simulink model "TosAeb"  (R2021a)
settings: Solver=FixedStepDiscrete  StartTime=0.0  StopTime=inf  FixedStep=0.01  RelTol=1e-3
undefined symbols (not defined in the file or in its folder): ACCModSts, AccSts1, AEB_ObjectMovingOrientation, AEB_ObjectMovingState, CN_BTCActive, CN_CurrentGearR, CN_CurrentGearR_DHT, CN_PTCActive, CN_VDCActive, CN_VSC_EspOff, CN_VSC_FCW_AEB_Error, CN_VSC_FCW_AEB_NoDispay, CN_VSC_FCW_AEB_PerfmDgdtn, CN_VSC_FCW_AEB_TempUnAvlbl, CN_VSC_VehStandstill, CNaccelAvoidMax, CNaccelAvoidMin, CNbrakePedalPress, CNcomfortStrLeftAccelMax, CNcomfortStrLeftAccelMin, CNcomfortStrRightAccelMax, CNcomfortStrRightAccelMin, CNDrvDoorOpen, CNDrvModeEco, CNDrvModeNorm, CNDrvModeOther, CNDrvModeSport, CNDrvSBRreminder, CNNull, CNobjInvalidID, CNPassDoorOpen, CNPassSBRreminder, CNPEBSAccelNegInf, CNPEBSAccelNull, CNPEBSTimeInf, CNPEBSTimeNull, CNSTOPMAX, CNSTOPNULL, CNsubjWidth, CNSwtOFF, CNSwtON, CNTTCMAX, CNTTCNULL, CNVehConfigAEBCycNone, CNVehConfigAEBNone, CNVehConfigAEBPedNone, CNVehConfigFCWNone, CNVehConfigJANone, CollisionSeverity, FALSE, FCWSnvty, JACycObjCalcInfoDefaultParams, JAPedObjCalcInfoDefaultParams, JAVehObjCalcInfoDefaultParams, latCycObjCalcInfoDefaultParams, latPedObjCalcInfoDefaultParams, longObjCalcInfoDefaultParams, ModSts, movingStatus, ObjectType ... (+1112)
libraries: simulink x3031, AEBSFunctionLib x50, Ta_Library x33, AEBSStateMachineLib x23, Mylibrary x12, simulink_extras x7, ObjSelect_lib x3, dspstat3 x2
(part 14 of 102)

== system TosAeb/FcwAebJa_Step_sys/ALG_AEBSystem/ALG_AEBController/AEB_FuncCondCalcApp/LongFunctionCondCalc/vehAEBfullSuppAborCondCalc ==
  inputSignals : Inport
  " vehAEBfullSuppCondCalc" : Subsystem
  vehAEBfullAborCondCalc : Subsystem
  vehAEBfullSuppCond : Outport
  vehAEBfullAborCondNoStandstill : Outport  Port=2
  vehAEBfullAborCondStandstill : Outport  Port=3
  wires:
  vehAEBfullAborCondCalc/2(vehAEBfullAborCondStandstillFulfilled) -> vehAEBfullAborCondStandstill/1  "vehAEBfullAborCondStandstill"
  " vehAEBfullSuppCondCalc"/1(vehAEBfullSuppCondFulfilled) -> vehAEBfullSuppCond/1  "vehAEBfullSuppCond"
  vehAEBfullAborCondCalc/1(vehAEBfullAborCondNoStandstillFulfilled) -> vehAEBfullAborCondNoStandstill/1  "vehAEBfullAborCondNoStandstill"
  inputSignals/1 -> " vehAEBfullSuppCondCalc"/1(inputSignals), vehAEBfullAborCondCalc/1(inputSignals)

== system TosAeb/FcwAebJa_Step_sys/ALG_AEBSystem/ALG_AEBController/AEB_FuncCondCalcApp/LongFunctionCondCalc/vehAEBpartSuppAborCondCalc ==
  inputSignals : Inport
  vehAEBpartAborCondCalc : Subsystem
  vehAEBpartSuppCondCalc : Subsystem
  vehAEBpartSuppCond : Outport
  vehAEBpartAborCondNoStandstill : Outport  Port=2
  vehAEBpartAborCondStandstill : Outport  Port=3
  wires:
  vehAEBpartSuppCondCalc/1(vehAEBpartSuppCondFulfilled) -> vehAEBpartSuppCond/1  "vehAEBpartSuppCond"
  vehAEBpartAborCondCalc/2(vehAEBpartAborCondStandstillFulfilled) -> vehAEBpartAborCondStandstill/1  "vehAEBpartAborCondStandstill"
  vehAEBpartAborCondCalc/1(vehAEBpartAborCondNoStandstillFulfilled) -> vehAEBpartAborCondNoStandstill/1  "vehAEBpartAborCondNoStandstill"
  inputSignals/1 -> vehAEBpartSuppCondCalc/1(inputSignals), vehAEBpartAborCondCalc/1(inputSignals)

== system TosAeb/FcwAebJa_Step_sys/ALG_AEBSystem/ALG_AEBController/AEB_FuncCondCalcApp/LongFunctionCondCalc/vehAcuteWarningSuppAborCondFulfilled ==
  inputSignals : Inport
  vehAcuteWarningAborCondCalc : Subsystem
  vehAcuteWarningSuppCondCalc : Subsystem
  vehAcuteWarningSuppCond : Outport
  vehAcuteWarningAborCond : Outport  Port=2
  wires:
  vehAcuteWarningSuppCondCalc/1(vehAcuteWarningSuppCond) -> vehAcuteWarningSuppCond/1  "vehAcuteWarningSuppCond"
  vehAcuteWarningAborCondCalc/1(vehAcuteWarningAborCond) -> vehAcuteWarningAborCond/1  "vehAcuteWarningAborCond"
  inputSignals/1 -> vehAcuteWarningSuppCondCalc/1(inputSignals), vehAcuteWarningAborCondCalc/1(inputSignals)

== system TosAeb/FcwAebJa_Step_sys/ALG_AEBSystem/ALG_AEBController/AEB_FuncCondCalcApp/LongFunctionCondCalc/vehEBASuppAborCondCalc ==
  inputSignals : Inport
  vehEBAAborCondCalc : Subsystem
  vehEBASuppCondCalc : Subsystem
  vehEBASuppCond : Outport
  vehEBAAborCondNoStandstill : Outport  Port=2
  vehEBAAborCondStandstill : Outport  Port=3
  wires:
  vehEBASuppCondCalc/1(vehEBASuppCondFulfilled) -> vehEBASuppCond/1  "vehEBASuppCond"
  vehEBAAborCondCalc/1(vehEBAAborCondNoStandstillFulfilled) -> vehEBAAborCondNoStandstill/1  "vehEBAAborCondNoStandstill"
  vehEBAAborCondCalc/2(vehEBAAborCondStandstillFulfilled) -> vehEBAAborCondStandstill/1  "vehEBAAborCondStandstill"
  inputSignals/1 -> vehEBASuppCondCalc/1(inputSignals), vehEBAAborCondCalc/1(inputSignals)

== system TosAeb/FcwAebJa_Step_sys/ALG_AEBSystem/ALG_AEBController/AEB_FuncCondCalcApp/LongFunctionCondCalc/vehLatentWarningSuppAborCondFulfilled ==
  inputSignals : Inport
  vehLatentWarningAborCondCalc : Subsystem
  vehLatentWarningSuppCondCalc : Subsystem
  vehLatentWarningSuppCond : Outport
  vehLatentWarningAborCond : Outport  Port=2
  wires:
  vehLatentWarningAborCondCalc/1(vehLatentWarningAborCondFulfilled) -> vehLatentWarningAborCond/1  "vehLatentWarningAborCond"
  vehLatentWarningSuppCondCalc/1(vehLatentWarningSuppCondFulfilled) -> vehLatentWarningSuppCond/1  "vehLatentWarningSuppCond"
  inputSignals/1 -> vehLatentWarningAborCondCalc/1(inputSignals), vehLatentWarningSuppCondCalc/1(inputSignals)

== system TosAeb/FcwAebJa_Step_sys/ALG_AEBSystem/ALG_AEBController/AEB_FuncCondCalcApp/LongFunctionCondCalc/vehPreWarningSuppAborCondFulfilled ==
  inputSignals : Inport
  vehPreWarningAborCondCalc : Subsystem
  vehPreWarningSuppCondCalc : Subsystem
  vehPreWarningSuppCond : Outport
  vehPreWarningAborCond : Outport  Port=2
  wires:
  inputSignals/1 -> vehPreWarningSuppCondCalc/1(inputSignals), vehPreWarningAborCondCalc/1(inputSignals)
  vehPreWarningSuppCondCalc/1(vehPreWarningSuppCond) -> vehPreWarningSuppCond/1  "vehPreWarningSuppCond"
  vehPreWarningAborCondCalc/1(vehPreWarningAborCond) -> vehPreWarningAborCond/1  "vehPreWarningAborCond"

== system TosAeb/FcwAebJa_Step_sys/ALG_AEBSystem/ALG_AEBController/AEB_FuncCondCalcApp/LongFunctionCondCalc/vehPrefillSuppAborCondCalc ==
  inputSignals : Inport
  vehPrefillAborCondCalc : Subsystem
  vehPrefillSuppCondCalc : Subsystem
  vehPrefillSuppCond : Outport
  vehPrefillAborCond : Outport  Port=2
  wires:
  vehPrefillAborCondCalc/1(vehPrefillAborCondFulfilled) -> vehPrefillAborCond/1  "vehPrefillAborCond"
  vehPrefillSuppCondCalc/1(vehPrefillSuppCondFulfilled) -> vehPrefillSuppCond/1  "vehPrefillSuppCond"
  inputSignals/1 -> vehPrefillSuppCondCalc/1(inputSignals), vehPrefillAborCondCalc/1(inputSignals)

== system TosAeb/FcwAebJa_Step_sys/ALG_AEBSystem/ALG_AEBController/AEB_FuncCondCalcApp/PedFunctionCondCalc/pedABASuppAborCond ==
  inputSignals : Inport
  pedABAAborCondCalc : Subsystem
  pedABASuppCondCalc : Subsystem
  pedABASuppCond : Outport
  pedABAAborCond : Outport  Port=2
  wires:
  inputSignals/1 -> pedABASuppCondCalc/1(inputSignals), pedABAAborCondCalc/1(inputSignals)
  pedABASuppCondCalc/1(pedABASuppCondFulfilled) -> pedABASuppCond/1  "pedPreWarningSuppCond"
  pedABAAborCondCalc/1(pedABAAborCondFulfilled) -> pedABAAborCond/1  "pedPreWarningAborCond"

== system TosAeb/FcwAebJa_Step_sys/ALG_AEBSystem/ALG_AEBController/AEB_FuncCondCalcApp/PedFunctionCondCalc/pedAEBfullSuppAborCond ==
  inputSignals : Inport
  pedAEBfullAborCondNoStandstillCalc : Subsystem
  pedAEBfullAborCondStandstillCalc : Subsystem
  pedAEBfullSuppCondCalc : Subsystem
  pedAEBfullSuppCond : Outport
  pedAEBfullAborCondNoStandstill : Outport  Port=2
  pedAEBfullAborCondStandstill : Outport  Port=3
  wires:
  pedAEBfullSuppCondCalc/1(pedAEBfullSuppCondFulfilled) -> pedAEBfullSuppCond/1  "pedAEBfullSuppCond"
  pedAEBfullAborCondNoStandstillCalc/1(pedAEBfullAborCondNoStandstillFulfilled) -> pedAEBfullAborCondNoStandstill/1  "pedAEBfullAborCondNoStandstill"
  pedAEBfullAborCondStandstillCalc/1(pedAEBfullAborCondStandstillFulfilled) -> pedAEBfullAborCondStandstill/1  "pedAEBfullAborCondStandstill"
  inputSignals/1 -> pedAEBfullAborCondStandstillCalc/1(inputSignals), pedAEBfullAborCondNoStandstillCalc/1(inputSignals), pedAEBfullSuppCondCalc/1(inputSignals)

== system TosAeb/FcwAebJa_Step_sys/ALG_AEBSystem/ALG_AEBController/AEB_FuncCondCalcApp/PedFunctionCondCalc/pedPreWarningSuppAborCond ==
  inputSignals : Inport
  pedPreWarningAborCondCalc : Subsystem
  pedPreWarningSuppCondCalc : Subsystem
  pedPreWarningSuppCond : Outport
  pedPreWarningAborCond : Outport  Port=2
  wires:
  pedPreWarningAborCondCalc/1(pedPreWarningAborCondFulfilled) -> pedPreWarningAborCond/1  "pedPreWarningAborCond"
  pedPreWarningSuppCondCalc/1(pedPreWarningSuppCondFulfilled) -> pedPreWarningSuppCond/1  "pedPreWarningSuppCond"
  inputSignals/1 -> pedPreWarningAborCondCalc/1(inputSignals), pedPreWarningSuppCondCalc/1(inputSignals)

== system TosAeb/FcwAebJa_Step_sys/ALG_AEBSystem/ALG_AEBController/AEB_FuncCondCalcApp/PedFunctionCondCalc/pedPrefillSuppAborCond ==
  inputSignals : Inport
  pedPrefillAborCondCalc : Subsystem
  pedPrefillSuppCondCalc : Subsystem
  pedPrefillSuppCond : Outport
  pedPrefillAborCond : Outport  Port=2
  wires:
  inputSignals/1 -> pedPrefillSuppCondCalc/1(inputSignals), pedPrefillAborCondCalc/1(inputSignals)
  pedPrefillAborCondCalc/1(pedPrefillAborCondFulfilled) -> pedPrefillAborCond/1  "pedPrefillAborCond"
  pedPrefillSuppCondCalc/1(pedPrefillSuppCondFulfilled) -> pedPrefillSuppCond/1  "pedPrefillSuppCond"

== system TosAeb/FcwAebJa_Step_sys/ALG_AEBSystem/ALG_AEBController/AEB_FuncCondCalcInfo_FUS/CycFunctionCondCalc/cycAEBfullSuppAborCond ==
  inputSignals : Inport
  Bus Selector : BusSelector  OutputSignals=FuncCondCalcInfo.CycFuncSuppAborCond.cycAEBfullSuppCond
  Bus Selector1 : BusSelector  OutputSignals=FuncCondCalcInfo.CycFuncSuppAborCond.cycAEBfullAborCondStandstill
  Bus Selector2 : BusSelector  OutputSignals=FuncCondCalcInfo.CycFuncSuppAborCond.cycAEBfullAborCondNoStandstill
  Constant : Constant
  Constant1 : Constant
  Constant2 : Constant
  Multiport Switch : MultiPortSwitch
  Multiport Switch1 : MultiPortSwitch
  Multiport Switch2 : MultiPortSwitch
  cycAEBfullSuppCond : Outport
  cycAEBfullAborCondNoStandstill : Outport  Port=2
  cycAEBfullAborCondStandstill : Outport  Port=3
  wires:
  Bus Selector1/1 -> Multiport Switch1/4, Multiport Switch1/3, Multiport Switch1/2  "<cycAEBfullAborCondStandstill>"
  Constant1/1 -> Multiport Switch1/1
  Constant/1 -> Multiport Switch/1
  Bus Selector2/1 -> Multiport Switch2/4, Multiport Switch2/3, Multiport Switch2/2  "<cycAEBfullAborCondNoStandstill>"
  Constant2/1 -> Multiport Switch2/1
  Bus Selector/1 -> Multiport Switch/4, Multiport Switch/3, Multiport Switch/2  "<cycAEBfullSuppCond>"
  inputSignals/1 -> Bus Selector/1, Bus Selector1/1, Bus Selector2/1
  Multiport Switch1/1 -> cycAEBfullAborCondStandstill/1  "cycAEBfullAborCondStandstill"
  Multiport Switch2/1 -> cycAEBfullAborCondNoStandstill/1  "cycAEBfullAborCondNoStandstill"
  Multiport Switch/1 -> cycAEBfullSuppCond/1  "cycAEBfullSuppCond"

== system TosAeb/FcwAebJa_Step_sys/ALG_AEBSystem/ALG_AEBController/AEB_FuncCondCalcInfo_FUS/CycFunctionCondCalc/cycAEBpartSuppAborCond ==
  inputSignals : Inport
  Bus Selector : BusSelector  OutputSignals=FuncCondCalcInfo.CycFuncSuppAborCond.cycAEBpartSuppCond
  Bus Selector1 : BusSelector  OutputSignals=FuncCondCalcInfo.CycFuncSuppAborCond.cycAEBpartAborCondStandstill
  Bus Selector2 : BusSelector  OutputSignals=FuncCondCalcInfo.CycFuncSuppAborCond.cycAEBpartAborCondNoStandstill
  Constant : Constant
  Constant1 : Constant
  Constant2 : Constant
  Multiport Switch : MultiPortSwitch
  Multiport Switch1 : MultiPortSwitch
  Multiport Switch2 : MultiPortSwitch
  cycAEBpartSuppCond : Outport
  cycAEBpartAborCondNoStandstill : Outport  Port=2
  cycAEBpartAborCondStandstill : Outport  Port=3
  wires:
  Bus Selector1/1 -> Multiport Switch1/4, Multiport Switch1/3, Multiport Switch1/2  "<cycAEBpartAborCondStandstill>"
  Constant1/1 -> Multiport Switch1/1
  Constant/1 -> Multiport Switch/1
  Bus Selector2/1 -> Multiport Switch2/4, Multiport Switch2/3, Multiport Switch2/2  "<cycAEBpartAborCondNoStandstill>"
  Constant2/1 -> Multiport Switch2/1
  Bus Selector/1 -> Multiport Switch/4, Multiport Switch/3, Multiport Switch/2  "<cycAEBpartSuppCond>"
  Multiport Switch2/1 -> cycAEBpartAborCondNoStandstill/1  "cycAEBpartAborCondNoStandstill"
  Multiport Switch1/1 -> cycAEBpartAborCondStandstill/1  "cycAEBpartAborCondStandstill"
  inputSignals/1 -> Bus Selector1/1, Bus Selector2/1, Bus Selector/1
  Multiport Switch/1 -> cycAEBpartSuppCond/1  "cycAEBpartSuppCond"

== system TosAeb/FcwAebJa_Step_sys/ALG_AEBSystem/ALG_AEBController/AEB_FuncCondCalcInfo_FUS/CycFunctionCondCalc/cycPrefillSuppAborCond ==
  inputSignals : Inport
  Bus Selector : BusSelector  OutputSignals=FuncCondCalcInfo.CycFuncSuppAborCond.cycPrefillSuppCond
  Bus Selector1 : BusSelector  OutputSignals=FuncCondCalcInfo.CycFuncSuppAborCond.cycPrefillAborCond
  Constant : Constant
  Constant2 : Constant
  Multiport Switch : MultiPortSwitch
  Multiport Switch1 : MultiPortSwitch
  cycPrefillSuppCond : Outport
  cycPrefillAborCond : Outport  Port=2
  wires:
  inputSignals/1 -> Bus Selector1/1, Bus Selector/1
  Bus Selector/1 -> Multiport Switch/4, Multiport Switch/3, Multiport Switch/2  "<cycPrefillSuppCond>"
  Constant/1 -> Multiport Switch/1
  Constant2/1 -> Multiport Switch1/1
  Bus Selector1/1 -> Multiport Switch1/4, Multiport Switch1/3, Multiport Switch1/2  "<cycPrefillAborCond>"
  Multiport Switch/1 -> cycPrefillSuppCond/1  "cycPrefillSuppCond"
  Multiport Switch1/1 -> cycPrefillAborCond/1  "cycPrefillAborCond"

== system TosAeb/FcwAebJa_Step_sys/ALG_AEBSystem/ALG_AEBController/AEB_FuncCondCalcInfo_FUS/JaCycFunctionCondCalc/JaCycAEBfullSuppAborCond ==
  inputSignals : Inport
  Bus Selector : BusSelector  OutputSignals=FuncCondCalcInfo.JACycFuncSuppAborCond.JACycAEBfullSuppCond
  Bus Selector1 : BusSelector  OutputSignals=FuncCondCalcInfo.JACycFuncSuppAborCond.JACycAEBfullAborCondStandstill
  Bus Selector2 : BusSelector  OutputSignals=FuncCondCalcInfo.JACycFuncSuppAborCond.JACycAEBfullAborCondNoStandstill
  Constant : Constant
  Constant1 : Constant
  Constant2 : Constant
  Multiport Switch : MultiPortSwitch
  Multiport Switch1 : MultiPortSwitch
  Multiport Switch2 : MultiPortSwitch
  JaCycAEBfullSuppCond : Outport
  JaCycAEBfullAborCondNoStandstill : Outport  Port=2
  JaCycAEBfullAborCondStandstill : Outport  Port=3
  wires:
  Multiport Switch1/1 -> JaCycAEBfullAborCondStandstill/1  "AEBJAPedAEBfullAborCondStandstill"
  inputSignals/1 -> Bus Selector1/1, Bus Selector2/1, Bus Selector/1
  Multiport Switch2/1 -> JaCycAEBfullAborCondNoStandstill/1  "AEBJAPedAEBfullAborCondNoStandstill"
  Multiport Switch/1 -> JaCycAEBfullSuppCond/1  "AEBJAPedAEBfullSuppCond"
  Bus Selector/1 -> Multiport Switch/2, Multiport Switch/4, Multiport Switch/3  "<JACycAEBfullSuppCond>"
  Constant2/1 -> Multiport Switch2/1
  Bus Selector2/1 -> Multiport Switch2/4, Multiport Switch2/3, Multiport Switch2/2  "<JACycAEBfullAborCondNoStandstill>"
  Constant/1 -> Multiport Switch/1
  Constant1/1 -> Multiport Switch1/1
  Bus Selector1/1 -> Multiport Switch1/4, Multiport Switch1/3, Multiport Switch1/2  "<JACycAEBfullAborCondStandstill>"

== system TosAeb/FcwAebJa_Step_sys/ALG_AEBSystem/ALG_AEBController/AEB_FuncCondCalcInfo_FUS/JaCycFunctionCondCalc/JaCycPrefillSuppAborCond ==
  inputSignals : Inport
  Bus Selector : BusSelector  OutputSignals=FuncCondCalcInfo.JACycFuncSuppAborCond.JACycPrefillSuppCond
  Bus Selector1 : BusSelector  OutputSignals=FuncCondCalcInfo.JACycFuncSuppAborCond.JACycPrefillAborCond
  Constant : Constant
  Constant1 : Constant
  Multiport Switch : MultiPortSwitch
  Multiport Switch1 : MultiPortSwitch
  JaCycPrefillSuppCond : Outport
  JaCycPrefillAborCond : Outport  Port=2
  wires:
  Multiport Switch/1 -> JaCycPrefillSuppCond/1
  Multiport Switch1/1 -> JaCycPrefillAborCond/1
  Bus Selector1/1 -> Multiport Switch1/4, Multiport Switch1/3, Multiport Switch1/2  "<JACycPrefillAborCond>"
  Bus Selector/1 -> Multiport Switch/4, Multiport Switch/3, Multiport Switch/2  "<JACycPrefillSuppCond>"
  Constant1/1 -> Multiport Switch1/1
  Constant/1 -> Multiport Switch/1
  inputSignals/1 -> Bus Selector1/1, Bus Selector/1

== system TosAeb/FcwAebJa_Step_sys/ALG_AEBSystem/ALG_AEBController/AEB_FuncCondCalcInfo_FUS/JaPedFunctionCondCalc/AEBJAPedAEBfullSuppAborCond ==
  inputSignals : Inport
  Bus Selector : BusSelector  OutputSignals=FuncCondCalcInfo.JAPedFuncSuppAborCond.JAPedAEBfullSuppCond
  Bus Selector1 : BusSelector  OutputSignals=FuncCondCalcInfo.JAPedFuncSuppAborCond.JAPedAEBfullAborCondStandstill
  Bus Selector2 : BusSelector  OutputSignals=FuncCondCalcInfo.JAPedFuncSuppAborCond.JAPedAEBfullAborCondNoStandstill
  Constant : Constant
  Constant1 : Constant
  Constant2 : Constant
  Multiport Switch : MultiPortSwitch
  Multiport Switch1 : MultiPortSwitch
  Multiport Switch2 : MultiPortSwitch
  AEBJAPedAEBfullSuppCond : Outport
  AEBJAPedAEBfullAborCondNoStandstill : Outport  Port=2
  AEBJAPedAEBfullAborCondStandstill : Outport  Port=3
  wires:
  Bus Selector1/1 -> Multiport Switch1/2, Multiport Switch1/4, Multiport Switch1/3  "<JAPedAEBfullAborCondStandstill>"
  Constant1/1 -> Multiport Switch1/1
  Constant/1 -> Multiport Switch/1
  Bus Selector2/1 -> Multiport Switch2/2, Multiport Switch2/4, Multiport Switch2/3  "<JAPedAEBfullAborCondNoStandstill>"
  Constant2/1 -> Multiport Switch2/1
  Bus Selector/1 -> Multiport Switch/4, Multiport Switch/3, Multiport Switch/2  "<JAPedAEBfullSuppCond>"
  Multiport Switch/1 -> AEBJAPedAEBfullSuppCond/1  "AEBJAPedAEBfullSuppCond"
  Multiport Switch2/1 -> AEBJAPedAEBfullAborCondNoStandstill/1  "AEBJAPedAEBfullAborCondNoStandstill"
  inputSignals/1 -> Bus Selector1/1, Bus Selector2/1, Bus Selector/1
  Multiport Switch1/1 -> AEBJAPedAEBfullAborCondStandstill/1  "AEBJAPedAEBfullAborCondStandstill"

== system TosAeb/FcwAebJa_Step_sys/ALG_AEBSystem/ALG_AEBController/AEB_FuncCondCalcInfo_FUS/JaPedFunctionCondCalc/AEBJAPedPrefillSuppAborCond ==
  inputSignals : Inport
  Bus Selector : BusSelector  OutputSignals=FuncCondCalcInfo.JAPedFuncSuppAborCond.JAPedPrefillSuppCond
  Bus Selector1 : BusSelector  OutputSignals=FuncCondCalcInfo.JAPedFuncSuppAborCond.JAPedPrefillAborCond
  Constant : Constant
  Constant1 : Constant
  Multiport Switch : MultiPortSwitch
  Multiport Switch1 : MultiPortSwitch
  JaPedPrefillSuppCond : Outport
  JaPedPrefillAborCond : Outport  Port=2
  wires:
  inputSignals/1 -> Bus Selector1/1, Bus Selector/1
  Constant/1 -> Multiport Switch/1
  Constant1/1 -> Multiport Switch1/1
  Bus Selector/1 -> Multiport Switch/4, Multiport Switch/3, Multiport Switch/2  "<JAPedPrefillSuppCond>"
  Bus Selector1/1 -> Multiport Switch1/4, Multiport Switch1/3, Multiport Switch1/2  "<JAPedPrefillAborCond>"
  Multiport Switch1/1 -> JaPedPrefillAborCond/1  "AEBJAPedPrefillAborCond"
  Multiport Switch/1 -> JaPedPrefillSuppCond/1  "AEBJAPedPrefillSuppCond"

== system TosAeb/FcwAebJa_Step_sys/ALG_AEBSystem/ALG_AEBController/AEB_FuncCondCalcInfo_FUS/JaVehFunctionCondCalc/JaVehAEBfullSuppAborCond ==
  inputSignals : Inport
  Bus Selector : BusSelector  OutputSignals=FuncCondCalcInfo.JAVehFuncSuppAborCond.JAVehAEBfullSuppCond
  Bus Selector1 : BusSelector  OutputSignals=FuncCondCalcInfo.JAVehFuncSuppAborCond.JAVehAEBfullAborCondStandstill
  Bus Selector2 : BusSelector  OutputSignals=FuncCondCalcInfo.JAVehFuncSuppAborCond.JAVehAEBfullAborCondNoStandstill
  Constant : Constant
  Constant1 : Constant
  Constant2 : Constant
  Multiport Switch : MultiPortSwitch
  Multiport Switch1 : MultiPortSwitch
  Multiport Switch2 : MultiPortSwitch
  JaVehAEBfullSuppCond : Outport
  JaVehAEBfullAborCondNoStandstill : Outport  Port=2
  JaVehAEBfullAborCondStandstill : Outport  Port=3
  wires:
  inputSignals/1 -> Bus Selector1/1, Bus Selector2/1, Bus Selector/1
  Constant/1 -> Multiport Switch/1
  Bus Selector/1 -> Multiport Switch/4, Multiport Switch/3, Multiport Switch/2  "<JAVehAEBfullSuppCond>"
  Constant2/1 -> Multiport Switch2/1
  Bus Selector2/1 -> Multiport Switch2/4, Multiport Switch2/3, Multiport Switch2/2  "<JAVehAEBfullAborCondNoStandstill>"
  Constant1/1 -> Multiport Switch1/1
  Bus Selector1/1 -> Multiport Switch1/4, Multiport Switch1/3, Multiport Switch1/2  "<JAVehAEBfullAborCondStandstill>"
  Multiport Switch1/1 -> JaVehAEBfullAborCondStandstill/1  "AEBJAVehAEBfullAborCondStandstill"
  Multiport Switch2/1 -> JaVehAEBfullAborCondNoStandstill/1  "AEBJAVehAEBfullAborCondNoStandstill"
  Multiport Switch/1 -> JaVehAEBfullSuppCond/1  "AEBJAVehAEBfullSuppCond"

== system TosAeb/FcwAebJa_Step_sys/ALG_AEBSystem/ALG_AEBController/AEB_FuncCondCalcInfo_FUS/JaVehFunctionCondCalc/JaVehPrefillSuppAborCond ==
  inputSignals : Inport
  Bus Selector : BusSelector  OutputSignals=FuncCondCalcInfo.JAVehFuncSuppAborCond.JAVehPrefillSuppCond
  Bus Selector1 : BusSelector  OutputSignals=FuncCondCalcInfo.JAVehFuncSuppAborCond.JAVehPrefillSuppCond
  Constant : Constant
  Constant1 : Constant
  Multiport Switch : MultiPortSwitch
  Multiport Switch1 : MultiPortSwitch
  JaVehPrefillSuppCond : Outport
  JaVehPrefillAborCond : Outport  Port=2
  wires:
  inputSignals/1 -> Bus Selector1/1, Bus Selector/1
  Constant/1 -> Multiport Switch/1
  Constant1/1 -> Multiport Switch1/1
  Bus Selector/1 -> Multiport Switch/4, Multiport Switch/3, Multiport Switch/2  "<JAVehPrefillSuppCond>"
  Bus Selector1/1 -> Multiport Switch1/4, Multiport Switch1/3, Multiport Switch1/2  "<JAVehPrefillSuppCond>"
  Multiport Switch/1 -> JaVehPrefillSuppCond/1  "AEBJAVehPrefillSuppCond"
  Multiport Switch1/1 -> JaVehPrefillAborCond/1  "AEBJAVehPrefillAborCond"

== system TosAeb/FcwAebJa_Step_sys/ALG_AEBSystem/ALG_AEBController/AEB_FuncCondCalcInfo_FUS/LongFunctionCondCalc/vehABASuppAborCondCalc ==
  inputSignals : Inport
  Bus Selector : BusSelector  OutputSignals=FuncCondCalcInfo.VehFuncSuppAborCond.vehABASuppCond
  Bus Selector1 : BusSelector  OutputSignals=FuncCondCalcInfo.VehFuncSuppAborCond.vehABAAborCond
  Constant : Constant
  Constant1 : Constant
  Multiport Switch : MultiPortSwitch
  Multiport Switch1 : MultiPortSwitch
  vehABASuppCond : Outport
  vehABAAborCond : Outport  Port=2
  wires:
  inputSignals/1 -> Bus Selector1/1, Bus Selector/1
  Constant/1 -> Multiport Switch/1
  Bus Selector/1 -> Multiport Switch/4, Multiport Switch/2, Multiport Switch/3  "<vehABASuppCond>"
  Bus Selector1/1 -> Multiport Switch1/4, Multiport Switch1/3, Multiport Switch1/2  "<vehABAAborCond>"
  Constant1/1 -> Multiport Switch1/1
  Multiport Switch1/1 -> vehABAAborCond/1  "vehABAAborCond"
  Multiport Switch/1 -> vehABASuppCond/1  "vehABASuppCond"

== system TosAeb/FcwAebJa_Step_sys/ALG_AEBSystem/ALG_AEBController/AEB_FuncCondCalcInfo_FUS/LongFunctionCondCalc/vehAEBfullSuppAborCondCalc ==
  inputSignals : Inport
  Bus Selector : BusSelector  OutputSignals=FuncCondCalcInfo.VehFuncSuppAborCond.vehAEBfullSuppCond
  Bus Selector1 : BusSelector  OutputSignals=FuncCondCalcInfo.VehFuncSuppAborCond.vehAEBfullAborCondStandstill
  Bus Selector2 : BusSelector  OutputSignals=FuncCondCalcInfo.VehFuncSuppAborCond.vehAEBfullAborCondNoStandstill
  Constant : Constant
  Constant1 : Constant
  Constant2 : Constant
  Multiport Switch : MultiPortSwitch
  Multiport Switch1 : MultiPortSwitch
  Multiport Switch2 : MultiPortSwitch
  vehAEBfullSuppCond : Outport
  vehAEBfullAborCondNoStandstill : Outport  Port=2
  vehAEBfullAborCondStandstill : Outport  Port=3
  wires:
  inputSignals/1 -> Bus Selector1/1, Bus Selector2/1, Bus Selector/1
  Bus Selector1/1 -> Multiport Switch1/2, Multiport Switch1/4, Multiport Switch1/3  "<vehAEBfullAborCondStandstill>"
  Constant2/1 -> Multiport Switch2/1
  Constant1/1 -> Multiport Switch1/1
  Bus Selector/1 -> Multiport Switch/4, Multiport Switch/3, Multiport Switch/2  "<vehAEBfullSuppCond>"
  Constant/1 -> Multiport Switch/1
  Bus Selector2/1 -> Multiport Switch2/4, Multiport Switch2/3, Multiport Switch2/2  "<vehAEBfullAborCondNoStandstill>"
  Multiport Switch1/1 -> vehAEBfullAborCondStandstill/1  "vehAEBfullAborCondStandstill"
  Multiport Switch/1 -> vehAEBfullSuppCond/1  "vehAEBfullSuppCond"
  Multiport Switch2/1 -> vehAEBfullAborCondNoStandstill/1  "vehAEBfullAborCondNoStandstill"

== system TosAeb/FcwAebJa_Step_sys/ALG_AEBSystem/ALG_AEBController/AEB_FuncCondCalcInfo_FUS/LongFunctionCondCalc/vehAEBpartSuppAborCondCalc ==
  inputSignals : Inport
  Bus Selector : BusSelector  OutputSignals=FuncCondCalcInfo.VehFuncSuppAborCond.vehAEBpartSuppCond
  Bus Selector1 : BusSelector  OutputSignals=FuncCondCalcInfo.VehFuncSuppAborCond.vehAEBpartAborCondStandstill
  Bus Selector2 : BusSelector  OutputSignals=FuncCondCalcInfo.VehFuncSuppAborCond.vehAEBpartAborCondNoStandstill
  Constant : Constant
  Constant1 : Constant
  Constant2 : Constant
  Multiport Switch : MultiPortSwitch
  Multiport Switch1 : MultiPortSwitch
  Multiport Switch2 : MultiPortSwitch
  vehAEBpartSuppCond : Outport
  vehAEBpartAborCondNoStandstill : Outport  Port=2
  vehAEBpartAborCondStandstill : Outport  Port=3
  wires:
  Constant2/1 -> Multiport Switch2/1
  inputSignals/1 -> Bus Selector1/1, Bus Selector2/1, Bus Selector/1
  Constant/1 -> Multiport Switch/1
  Bus Selector/1 -> Multiport Switch/4, Multiport Switch/3, Multiport Switch/2  "<vehAEBpartSuppCond>"
  Bus Selector1/1 -> Multiport Switch1/4, Multiport Switch1/3, Multiport Switch1/2  "<vehAEBpartAborCondStandstill>"
  Constant1/1 -> Multiport Switch1/1
  Multiport Switch/1 -> vehAEBpartSuppCond/1  "vehAEBpartSuppCond"
  Multiport Switch1/1 -> vehAEBpartAborCondStandstill/1  "vehAEBpartAborCondStandstill"
  Multiport Switch2/1 -> vehAEBpartAborCondNoStandstill/1  "vehAEBpartAborCondNoStandstill"
  Bus Selector2/1 -> Multiport Switch2/4, Multiport Switch2/3, Multiport Switch2/2  "<vehAEBpartAborCondNoStandstill>"

== system TosAeb/FcwAebJa_Step_sys/ALG_AEBSystem/ALG_AEBController/AEB_FuncCondCalcInfo_FUS/LongFunctionCondCalc/vehAcuteWarningSuppAborCondFulfilled ==
  inputSignals : Inport
  Bus Selector : BusSelector  OutputSignals=FuncCondCalcInfo.VehFuncSuppAborCond.vehAcuteWarningSuppCond
  Bus Selector1 : BusSelector  OutputSignals=FuncCondCalcInfo.VehFuncSuppAborCond.vehAcuteWarningAborCond
  Constant : Constant
  Constant1 : Constant
  Multiport Switch : MultiPortSwitch
  Multiport Switch1 : MultiPortSwitch
  vehAcuteWarningSuppCond : Outport
  vehAcuteWarningAborCond : Outport  Port=2
  wires:
  Bus Selector/1 -> Multiport Switch/4, Multiport Switch/3, Multiport Switch/2  "<vehAcuteWarningSuppCond>"
  inputSignals/1 -> Bus Selector1/1, Bus Selector/1
  Constant/1 -> Multiport Switch/1
  Bus Selector1/1 -> Multiport Switch1/4, Multiport Switch1/3, Multiport Switch1/2  "<vehAcuteWarningAborCond>"
  Constant1/1 -> Multiport Switch1/1
  Multiport Switch/1 -> vehAcuteWarningSuppCond/1  "vehAcuteWarningSuppCond"
  Multiport Switch1/1 -> vehAcuteWarningAborCond/1  "vehAcuteWarningAborCond"

== system TosAeb/FcwAebJa_Step_sys/ALG_AEBSystem/ALG_AEBController/AEB_FuncCondCalcInfo_FUS/LongFunctionCondCalc/vehEBASuppAborCondCalc ==
  inputSignals : Inport
  Bus Selector : BusSelector  OutputSignals=FuncCondCalcInfo.VehFuncSuppAborCond.vehEBASuppCond
  Bus Selector1 : BusSelector  OutputSignals=FuncCondCalcInfo.VehFuncSuppAborCond.vehEBAAborCondStandstill
  Bus Selector2 : BusSelector  OutputSignals=FuncCondCalcInfo.VehFuncSuppAborCond.vehEBAAborCondNoStandstill
  Constant : Constant
  Constant1 : Constant
  Constant2 : Constant
  Multiport Switch : MultiPortSwitch
  Multiport Switch1 : MultiPortSwitch
  Multiport Switch2 : MultiPortSwitch
  vehEBASuppCond : Outport
  vehEBAAborCondNoStandstill : Outport  Port=2
  vehEBAAborCondStandstill : Outport  Port=3
  wires:
  inputSignals/1 -> Bus Selector1/1, Bus Selector2/1, Bus Selector/1
  Bus Selector1/1 -> Multiport Switch1/4, Multiport Switch1/3, Multiport Switch1/2  "<vehEBAAborCondStandstill>"
  Constant2/1 -> Multiport Switch2/1
  Constant1/1 -> Multiport Switch1/1
  Bus Selector/1 -> Multiport Switch/4, Multiport Switch/3, Multiport Switch/2  "<vehEBASuppCond>"
  Constant/1 -> Multiport Switch/1
  Bus Selector2/1 -> Multiport Switch2/4, Multiport Switch2/3, Multiport Switch2/2  "<vehEBAAborCondNoStandstill>"
  Multiport Switch/1 -> vehEBASuppCond/1  "vehEBASuppCond"
  Multiport Switch2/1 -> vehEBAAborCondNoStandstill/1  "vehEBAAborCondNoStandstill"
  Multiport Switch1/1 -> vehEBAAborCondStandstill/1  "vehEBAAborCondStandstill"

== system TosAeb/FcwAebJa_Step_sys/ALG_AEBSystem/ALG_AEBController/AEB_FuncCondCalcInfo_FUS/LongFunctionCondCalc/vehLatentWarningSuppAborCondFulfilled ==
  inputSignals : Inport
  Bus Selector : BusSelector  OutputSignals=FuncCondCalcInfo.VehFuncSuppAborCond.vehLatentWarningSuppCond,FuncCondCalcInfo.VehFuncSuppAborCond.vehLatentWarningAborCond
  vehLatentWarningSuppCond : Outport
  vehLatentWarningAborCond : Outport  Port=2
  wires:
  inputSignals/1 -> Bus Selector/1
  Bus Selector/2 -> vehLatentWarningAborCond/1  "<vehLatentWarningAborCond>"
  Bus Selector/1 -> vehLatentWarningSuppCond/1  "<vehLatentWarningSuppCond>"

== system TosAeb/FcwAebJa_Step_sys/ALG_AEBSystem/ALG_AEBController/AEB_FuncCondCalcInfo_FUS/LongFunctionCondCalc/vehPreWarningSuppAborCondFulfilled ==
  note: Lon_FCW_Config_Set
  inputSignals : Inport
  Bus Selector : BusSelector  OutputSignals=FuncCondCalcInfo.VehFuncSuppAborCond.vehPreWarningSuppCond
  Bus Selector1 : BusSelector  OutputSignals=FuncCondCalcInfo.VehFuncSuppAborCond.vehPreWarningAborCond
  Constant : Constant
  Constant1 : Constant
  Multiport Switch : MultiPortSwitch
  Multiport Switch1 : MultiPortSwitch
  vehPreWarningSuppCond : Outport
  vehPreWarningAborCond : Outport  Port=2
  wires:
  Bus Selector1/1 -> Multiport Switch1/4, Multiport Switch1/3, Multiport Switch1/2  "<vehPreWarningAborCond>"
  Constant1/1 -> Multiport Switch1/1
  Constant/1 -> Multiport Switch/1
  Bus Selector/1 -> Multiport Switch/4, Multiport Switch/2, Multiport Switch/3  "<vehPreWarningSuppCond>"
  inputSignals/1 -> Bus Selector1/1, Bus Selector/1
  Multiport Switch/1 -> vehPreWarningSuppCond/1  "vehPreWarningSuppCond"
  Multiport Switch1/1 -> vehPreWarningAborCond/1  "vehPreWarningAborCond"

== system TosAeb/FcwAebJa_Step_sys/ALG_AEBSystem/ALG_AEBController/AEB_FuncCondCalcInfo_FUS/LongFunctionCondCalc/vehPrefillSuppAborCondCalc ==
  inputSignals : Inport
  Bus Selector : BusSelector  OutputSignals=FuncCondCalcInfo.VehFuncSuppAborCond.vehPrefillSuppCond
  Bus Selector1 : BusSelector  OutputSignals=FuncCondCalcInfo.VehFuncSuppAborCond.vehPrefillAborCond
  Constant : Constant
  Constant1 : Constant
  Multiport Switch : MultiPortSwitch
  Multiport Switch1 : MultiPortSwitch
  vehPrefillSuppCond : Outport
  vehPrefillAborCond : Outport  Port=2
  wires:
  inputSignals/1 -> Bus Selector1/1, Bus Selector/1
  Constant/1 -> Multiport Switch/1
  Bus Selector/1 -> Multiport Switch/4, Multiport Switch/3, Multiport Switch/2  "<vehPrefillSuppCond>"
  Bus Selector1/1 -> Multiport Switch1/4, Multiport Switch1/3, Multiport Switch1/2  "<vehPrefillAborCond>"
  Constant1/1 -> Multiport Switch1/1
  Multiport Switch1/1 -> vehPrefillAborCond/1  "vehPrefillAborCond"
  Multiport Switch/1 -> vehPrefillSuppCond/1  "vehPrefillSuppCond"

== system TosAeb/FcwAebJa_Step_sys/ALG_AEBSystem/ALG_AEBController/AEB_FuncCondCalcInfo_FUS/PedFunctionCondCalc/pedABASuppAborCond ==
  inputSignals : Inport
  Bus Selector : BusSelector  OutputSignals=FuncCondCalcInfo.PedFuncSuppAborCond.pedABASuppCond
  Bus Selector1 : BusSelector  OutputSignals=FuncCondCalcInfo.PedFuncSuppAborCond.pedABAAborCond
  Constant : Constant
  Constant1 : Constant
  Multiport Switch : MultiPortSwitch
  Multiport Switch1 : MultiPortSwitch
  pedABASuppCond : Outport
  pedABAAborCond : Outport  Port=2
  wires:
  inputSignals/1 -> Bus Selector1/1, Bus Selector/1
  Constant1/1 -> Multiport Switch1/1
  Bus Selector1/1 -> Multiport Switch1/4, Multiport Switch1/3, Multiport Switch1/2  "<pedABAAborCond>"
  Bus Selector/1 -> Multiport Switch/4, Multiport Switch/3, Multiport Switch/2  "<pedABASuppCond>"
  Constant/1 -> Multiport Switch/1
  Multiport Switch/1 -> pedABASuppCond/1  "pedABASuppCond"
  Multiport Switch1/1 -> pedABAAborCond/1  "pedABAAborCond"

== system TosAeb/FcwAebJa_Step_sys/ALG_AEBSystem/ALG_AEBController/AEB_FuncCondCalcInfo_FUS/PedFunctionCondCalc/pedAEBfullSuppAborCond ==
  inputSignals : Inport
  Bus Selector : BusSelector  OutputSignals=FuncCondCalcInfo.PedFuncSuppAborCond.pedAEBfullSuppCond
  Bus Selector1 : BusSelector  OutputSignals=FuncCondCalcInfo.PedFuncSuppAborCond.pedAEBfullAborCondStandstill
  Bus Selector2 : BusSelector  OutputSignals=FuncCondCalcInfo.PedFuncSuppAborCond.pedAEBfullAborCondNoStandstill
  Constant : Constant
  Constant1 : Constant
  Constant2 : Constant
  Multiport Switch : MultiPortSwitch
  Multiport Switch1 : MultiPortSwitch
  Multiport Switch2 : MultiPortSwitch
  pedAEBfullSuppCond : Outport
  pedAEBfullAborCondNoStandstill : Outport  Port=2
  pedAEBfullAborCondStandstill : Outport  Port=3
  wires:
  inputSignals/1 -> Bus Selector1/1, Bus Selector2/1, Bus Selector/1
  Bus Selector/1 -> Multiport Switch/4, Multiport Switch/3, Multiport Switch/2  "<pedAEBfullSuppCond>"
  Constant2/1 -> Multiport Switch2/1
  Bus Selector2/1 -> Multiport Switch2/4, Multiport Switch2/3, Multiport Switch2/2  "<pedAEBfullAborCondNoStandstill>"
  Constant/1 -> Multiport Switch/1
  Constant1/1 -> Multiport Switch1/1
  Bus Selector1/1 -> Multiport Switch1/4, Multiport Switch1/3, Multiport Switch1/2  "<pedAEBfullAborCondStandstill>"
  Multiport Switch/1 -> pedAEBfullSuppCond/1  "pedAEBfullSuppCond"
  Multiport Switch2/1 -> pedAEBfullAborCondNoStandstill/1  "pedAEBfullAborCondNoStandstill"
  Multiport Switch1/1 -> pedAEBfullAborCondStandstill/1  "pedAEBfullAborCondStandstill"

== system TosAeb/FcwAebJa_Step_sys/ALG_AEBSystem/ALG_AEBController/AEB_FuncCondCalcInfo_FUS/PedFunctionCondCalc/pedPreWarningSuppAborCond ==
  inputSignals : Inport
  Bus Selector : BusSelector  OutputSignals=FuncCondCalcInfo.PedFuncSuppAborCond.pedPreWarningSuppCond
  Bus Selector1 : BusSelector  OutputSignals=FuncCondCalcInfo.PedFuncSuppAborCond.pedPreWarningAborCond
  Constant : Constant
  Constant1 : Constant
  Multiport Switch : MultiPortSwitch
  Multiport Switch1 : MultiPortSwitch
  pedPreWarningSuppCond : Outport
  pedPreWarningAborCond : Outport  Port=2
  wires:
  inputSignals/1 -> Bus Selector1/1, Bus Selector/1
  Constant1/1 -> Multiport Switch1/1
  Bus Selector1/1 -> Multiport Switch1/4, Multiport Switch1/3, Multiport Switch1/2  "<pedPreWarningAborCond>"
  Bus Selector/1 -> Multiport Switch/4, Multiport Switch/3, Multiport Switch/2  "<pedPreWarningSuppCond>"
  Constant/1 -> Multiport Switch/1
  Multiport Switch1/1 -> pedPreWarningAborCond/1  "pedPreWarningAborCond"
  Multiport Switch/1 -> pedPreWarningSuppCond/1  "pedPreWarningSuppCond"

== system TosAeb/FcwAebJa_Step_sys/ALG_AEBSystem/ALG_AEBController/AEB_FuncCondCalcInfo_FUS/PedFunctionCondCalc/pedPrefillSuppAborCond ==
  inputSignals : Inport
  Bus Selector : BusSelector  OutputSignals=FuncCondCalcInfo.PedFuncSuppAborCond.pedPrefillSuppCond
  Bus Selector1 : BusSelector  OutputSignals=FuncCondCalcInfo.PedFuncSuppAborCond.pedPrefillAborCond
  Constant : Constant
  Constant1 : Constant
  Multiport Switch : MultiPortSwitch
  Multiport Switch1 : MultiPortSwitch
  pedPrefillSuppCond : Outport
  pedPrefillAborCond : Outport  Port=2
  wires:
  Constant/1 -> Multiport Switch/1
  Bus Selector/1 -> Multiport Switch/4, Multiport Switch/3, Multiport Switch/2  "<pedPrefillSuppCond>"
  Bus Selector1/1 -> Multiport Switch1/4, Multiport Switch1/3, Multiport Switch1/2  "<pedPrefillAborCond>"
  Constant1/1 -> Multiport Switch1/1
  inputSignals/1 -> Bus Selector1/1, Bus Selector/1
  Multiport Switch1/1 -> pedPrefillAborCond/1  "pedPrefillAborCond"
  Multiport Switch/1 -> pedPrefillSuppCond/1  "pedPrefillSuppCond"

== system TosAeb/FcwAebJa_Step_sys/ALG_AEBSystem/ALG_AEBController/AEB_FuncImpManagementApp/FuncBlockTimeCalc/tBlockTimeBrkCalc ==
  AEBModStsFuncImpArb : Inport  OutDataTypeStr=Bus: BusAEBModStsFuncImpArb
  BlockTimeCalc : Subsystem
  blockTimeTriggerCondCalc : Subsystem
  tBlockTimeBrk : Outport
  wires:
  BlockTimeCalc/1(blockTime) -> tBlockTimeBrk/1
  blockTimeTriggerCondCalc/1(blockTimeTriggerCond) -> BlockTimeCalc/1(blockTimeTriggerCond)
  AEBModStsFuncImpArb/1 -> blockTimeTriggerCondCalc/1(AEBModStsFuncImpArb)

== system TosAeb/FcwAebJa_Step_sys/ALG_AEBSystem/ALG_AEBController/AEB_FuncImpManagementApp/FuncBlockTimeCalc/tBlockTimeBrkReadyCalc ==
  AEBModStsFuncImpArb : Inport  OutDataTypeStr=Bus: BusAEBModStsFuncImpArb
  BlockTimeCalc : Subsystem
  blockTimeTriggerCondCalc : Subsystem
  tBlockTimeBrkReady : Outport
  wires:
  BlockTimeCalc/1(blockTime) -> tBlockTimeBrkReady/1
  blockTimeTriggerCondCalc/1(blockTimeTriggerCond) -> BlockTimeCalc/1(blockTimeTriggerCond)
  AEBModStsFuncImpArb/1 -> blockTimeTriggerCondCalc/1(AEBModStsFuncImpArb)

== system TosAeb/FcwAebJa_Step_sys/ALG_AEBSystem/ALG_AEBController/AEB_FuncImpManagementApp/FuncBlockTimeCalc/tBlockTimeWarningCalc ==
  AEBModStsFuncImpArb : Inport  OutDataTypeStr=Bus: BusAEBModStsFuncImpArb
  BlockTimeCalc : Subsystem
  blockTimeTriggerCondCalc : Subsystem
  tBlockTimeWarning : Outport
  wires:
  AEBModStsFuncImpArb/1 -> blockTimeTriggerCondCalc/1(AEBModStsFuncImpArb)
  blockTimeTriggerCondCalc/1(blockTimeTriggerCond) -> BlockTimeCalc/1(blockTimeTriggerCond)
  BlockTimeCalc/1(blockTime) -> tBlockTimeWarning/1

== system TosAeb/FcwAebJa_Step_sys/ALG_AEBSystem/ALG_AEBController/AEB_FuncImpManagementApp/FuncImpArbCalc/BrkFuncImpArbCalc ==
  AEBpartModStsArb : Inport
  AEBfullModStsArb : Inport  Port=2
  EBAModStsArb : Inport  Port=3
  cycAEBpartModStsArb : Inport  Port=4
  cycAEBfullModStsArb : Inport  Port=5
  pedAEBfullModStsArb : Inport  Port=6
  JA_vehAEBModStsArb : Inport  Port=7
  JA_PTW_AEBModStsArb : Inport  Port=8
  JA_cycAEBModStsArb : Inport  Port=9
  JA_pedAEBModStsArb : Inport  Port=10
  JA_pedAEBfullModStsArb : Inport  Port=11  OutDataTypeStr=Enum: ModSts
  JA_cycAEBfullModStsArb : Inport  Port=12  OutDataTypeStr=Enum: ModSts
  JA_PTW_AEBfullModStsArb : Inport  Port=13  OutDataTypeStr=Enum: ModSts
  FCTBModStsArb : Inport  Port=14
  BrkFumcActiveCondCalc : Subsystem
  BrkFuncImpAborCondCalc : Subsystem
  BrkFuncImpAborCondCalc1 : Subsystem  Commented=on
  BrkFuncImpArbCalc : Subsystem
  BrkFuncImpSuppCondCalc : Subsystem
  BrkFuncImpSuppCondCalc1 : Subsystem  Commented=on
  Bus Creator : BusCreator  Inputs=11
  Bus Creator1 : BusCreator  Inputs=11
  Bus Creator2 : BusCreator  Inputs=2
  Bus Creator4 : BusCreator  Inputs=13
  Bus Creator5 : BusCreator  Inputs=11
  Bus Selector : BusSelector  OutputSignals=AEBpartFuncImpArb,cycAEBpartFuncImpArb,AEBfullFuncImpArb,JA_AEBfullFuncImpArb,cycAEBfullFuncImpArb,pedAEBfullFuncImpArb,EBAFuncImpArb,FCTBFuncImpArb,JA_PTW_AEBFuncImpArb,JA_cycAEBFuncImpArb,JA_pedAEBFuncImpArb
  Bus Selector1 : BusSelector  OutputSignals=AEBpartModStsArb,AEBfullModStsArb,EBAModStsArb,cycAEBpartModStsArb,cycAEBfullModStsArb,pedAEBfullModStsArb,JA_vehAEBModStsArb,JA_PTW_AEBModStsArb,JA_cycAEBModStsArb,JA_pedAEBModStsArb
  Bus Selector2 : BusSelector  OutputSignals=AEBpartModStsArb,AEBfullModStsArb,EBAModStsArb,cycAEBpartModStsArb,cycAEBfullModStsArb,pedAEBfullModStsArb,JA_vehAEBModStsArb,JA_PTW_AEBModStsArb,JA_cycAEBModStsArb,JA_pedAEBModStsArb
  brkFuncActvCond : Outport
  brkFuncImpArb : Outport  Port=2
  brkFuncImpSuppCondAbor : Outport  Port=3
  wires:
  Bus Selector/11 -> BrkFumcActiveCondCalc/11(JA_pedAEBFuncImpArb)  "<JA_pedAEBFuncImpArb>"
  Bus Selector/10 -> BrkFumcActiveCondCalc/10(JA_cycAEBFuncImpArb)  "<JA_cycAEBFuncImpArb>"
  Bus Selector/9 -> BrkFumcActiveCondCalc/9(JA_PTW_AEBFuncImpArb)  "<JA_PTW_AEBFuncImpArb>"
  BrkFuncImpArbCalc/11(JA_pedAEBFuncImpArb) -> Bus Creator5/11  "JA_pedAEBFuncImpArb"
  BrkFuncImpArbCalc/10(JA_cycAEBFuncImpArb) -> Bus Creator5/10  "JA_cycAEBFuncImpArb"
  BrkFuncImpArbCalc/9(JA_PTW_AEBFuncImpArb) -> Bus Creator5/9  "JA_PTW_AEBFuncImpArb"
  FCTBModStsArb/1 -> BrkFuncImpArbCalc/3(FCTBModStsArb)
  JA_PTW_AEBfullModStsArb/1 -> Bus Creator4/13  "JA_PTW_AEBfullModStsArb"
  JA_cycAEBfullModStsArb/1 -> Bus Creator4/12  "JA_cycAEBfullModStsArb"
  JA_pedAEBfullModStsArb/1 -> Bus Creator4/11  "JA_pedAEBfullModStsArb"
  JA_pedAEBModStsArb/1 -> Bus Creator4/10  "JA_pedAEBModStsArb"
  cycAEBfullModStsArb/1 -> Bus Creator4/5  "cycAEBfullModStsArb"
  cycAEBpartModStsArb/1 -> Bus Creator4/4  "cycAEBpartModStsArb"
  pedAEBfullModStsArb/1 -> Bus Creator4/6  "pedAEBfullModStsArb"
  AEBfullModStsArb/1 -> Bus Creator4/2  "AEBfullModStsArb"
  EBAModStsArb/1 -> Bus Creator4/3  "EBAModStsArb"
  JA_cycAEBModStsArb/1 -> Bus Creator4/9  "JA_cycAEBModStsArb"
  JA_PTW_AEBModStsArb/1 -> Bus Creator4/8  "JA_PTW_AEBModStsArb"
  AEBpartModStsArb/1 -> Bus Creator4/1  "AEBpartModStsArb"
  JA_vehAEBModStsArb/1 -> Bus Creator4/7  "JA_vehAEBModStsArb"
  BrkFuncImpSuppCondCalc/5(AEBfullFuncImpSuppCond) -> Bus Creator1/5  "vehAEBfullFuncImpSuppCond"
  BrkFuncImpSuppCondCalc/6(cycAEBfullFuncImpSuppCond) -> Bus Creator1/6  "cycAEBfullFuncImpSuppCond"
  BrkFuncImpSuppCondCalc/11(JA_PTW_AEBfullFuncImpSuppCond) -> Bus Creator1/11  "JA_PTW_AEBfullFuncImpSuppCond"
  BrkFuncImpSuppCondCalc/3(cycAEBpartFuncImpSuppCond) -> Bus Creator1/3  "cycAEBpartFuncImpSuppCond"
  BrkFuncImpSuppCondCalc/4(JA_vehAEBfullFuncImpSuppCond) -> Bus Creator1/4  "JA_vehAEBfullFuncImpSuppCond"
  BrkFuncImpSuppCondCalc/1(FCTBFuncImpSuppCond) -> Bus Creator1/1  "FCTBFuncImpSuppCond"
  BrkFuncImpSuppCondCalc/8(EBAFuncImpSuppCond) -> Bus Creator1/8  "vehEBAFuncImpSuppCond"
  BrkFuncImpSuppCondCalc/2(AEBpartFuncImpSuppCond) -> Bus Creator1/2  "vehAEBpartFuncImpSuppCond"
  BrkFuncImpSuppCondCalc/7(pedAEBfullFuncImpSuppCond) -> Bus Creator1/7  "pedAEBfullFuncImpSuppCond"
  BrkFuncImpSuppCondCalc/10(JA_cycAEBfullFuncImpSuppCond) -> Bus Creator1/10  "JA_cycAEBfullFuncImpSuppCond"
  BrkFuncImpSuppCondCalc/9(JA_pedAEBfullFuncImpSuppCond) -> Bus Creator1/9  "JA_pedAEBfullFuncImpSuppCond"
  Bus Selector2/1 -> BrkFuncImpSuppCondCalc/1(AEBpartModStsArb)  "<AEBpartModStsArb>"
  Bus Selector2/2 -> BrkFuncImpSuppCondCalc/2(AEBfullModStsArb)  "<AEBfullModStsArb>"
  Bus Selector2/3 -> BrkFuncImpSuppCondCalc/3(EBAModStsArb)  "<EBAModStsArb>"
  Bus Selector2/4 -> BrkFuncImpSuppCondCalc/4(cycAEBpartModStsArb)  "<cycAEBpartModStsArb>"
  Bus Selector2/5 -> BrkFuncImpSuppCondCalc/5(cycAEBfullModStsArb)  "<cycAEBfullModStsArb>"
  Bus Selector2/6 -> BrkFuncImpSuppCondCalc/6(pedAEBfullModStsArb)  "<pedAEBfullModStsArb>"
  Bus Selector2/7 -> BrkFuncImpSuppCondCalc/7(JA_vehAEBModStsArb)  "<JA_vehAEBModStsArb>"
  Bus Selector2/8 -> BrkFuncImpSuppCondCalc/8(JA_PTW_AEBModStsArb)  "<JA_PTW_AEBModStsArb>"
  Bus Selector2/9 -> BrkFuncImpSuppCondCalc/9(JA_cycAEBModStsArb)  "<JA_cycAEBModStsArb>"
  Bus Selector2/10 -> BrkFuncImpSuppCondCalc/10(JA_pedAEBModStsArb)  "<JA_pedAEBModStsArb>"
  BrkFuncImpAborCondCalc/11(JA_PTW_AEBfullFuncImpAborCond) -> Bus Creator/11  "JA_PTW_AEBfullFuncImpAborCond"
  BrkFuncImpAborCondCalc/10(JA_cycAEBfullFuncImpAborCond) -> Bus Creator/10  "JA_cycAEBfullFuncImpAborCond"
  BrkFuncImpAborCondCalc/9(JA_pedAEBfullFuncImpAborCond) -> Bus Creator/9  "JA_pedAEBfullFuncImpAborCond"
  Bus Selector1/10 -> BrkFuncImpAborCondCalc/10(JA_pedAEBModStsArb)  "<JA_pedAEBModStsArb>"
  Bus Selector1/9 -> BrkFuncImpAborCondCalc/9(JA_cycAEBModStsArb)  "<JA_cycAEBModStsArb>"
  Bus Selector1/8 -> BrkFuncImpAborCondCalc/8(JA_PTW_AEBModStsArb)  "<JA_PTW_AEBModStsArb>"
  Bus Selector1/7 -> BrkFuncImpAborCondCalc/7(JA_vehAEBModStsArb)  "<JA_vehAEBModStsArb>"
  Bus Selector1/6 -> BrkFuncImpAborCondCalc/6(pedAEBfullModStsArb)  "<pedAEBfullModStsArb>"
  Bus Selector1/5 -> BrkFuncImpAborCondCalc/5(cycAEBfullModStsArb)  "<cycAEBfullModStsArb>"
  Bus Selector1/4 -> BrkFuncImpAborCondCalc/4(cycAEBpartModStsArb)  "<cycAEBpartModStsArb>"
  Bus Selector1/3 -> BrkFuncImpAborCondCalc/3(EBAModStsArb)  "<EBAModStsArb>"
  Bus Selector1/2 -> BrkFuncImpAborCondCalc/2(AEBfullModStsArb)  "<AEBfullModStsArb>"
  Bus Selector1/1 -> BrkFuncImpAborCondCalc/1(AEBpartModStsArb)  "<AEBpartModStsArb>"
  BrkFuncImpAborCondCalc/6(cycAEBfullFuncImpAborCond) -> Bus Creator/6  "cycAEBfullFuncImpAborCond"
  BrkFuncImpAborCondCalc/1(FCTBFuncImpAborCond) -> Bus Creator/1  "FCTBFuncImpAborCond"
  BrkFuncImpAborCondCalc/8(EBAFuncImpAborCond) -> Bus Creator/8  "vehEBAFuncImpAborCond"
  BrkFuncImpAborCondCalc/4(JA_vehAEBfullFuncImpAborCond) -> Bus Creator/4  "JA_vehAEBfullFuncImpAborCond"
  BrkFuncImpAborCondCalc/2(AEBpartFuncImpAborCond) -> Bus Creator/2  "vehAEBpartFuncImpAborCond"
  BrkFuncImpAborCondCalc/3(cycAEBpartFuncImpAborCond) -> Bus Creator/3  "cycAEBpartFuncImpAborCond"
  BrkFuncImpAborCondCalc/7(pedAEBfullFuncImpAborCond) -> Bus Creator/7  "pedAEBfullFuncImpAborCond"
  BrkFuncImpAborCondCalc/5(AEBfullFuncImpAborCond) -> Bus Creator/5  "vehAEBfullFuncImpAborCond"
  Bus Creator5/1 -> brkFuncImpArb/1, Bus Selector/1  "brkFuncImpArb"
  Bus Creator2/1 -> brkFuncImpSuppCondAbor/1  "brkFuncImpSuppAbor"
  Bus Creator1/1 -> Bus Creator2/2  "brkFuncImpSuppCond"
  Bus Selector/6 -> BrkFumcActiveCondCalc/6(pedAEBfullFuncImpArb)  "<pedAEBfullFuncImpArb>"
  Bus Selector/7 -> BrkFumcActiveCondCalc/7(EBAFuncImpArb)  "<EBAFuncImpArb>"
  Bus Selector/3 -> BrkFumcActiveCondCalc/3(AEBfullFuncImpArb)  "<AEBfullFuncImpArb>"
  Bus Selector/2 -> BrkFumcActiveCondCalc/2(cycAEBpartFuncImpArb)  "<cycAEBpartFuncImpArb>"
  Bus Selector/5 -> BrkFumcActiveCondCalc/5(cycAEBfullFuncImpArb)  "<cycAEBfullFuncImpArb>"
  Bus Selector/8 -> BrkFumcActiveCondCalc/8(FCTBFuncImpArb)  "<FCTBFuncImpArb>"
  Bus Selector/4 -> BrkFumcActiveCondCalc/4(JA_vehAEBfullFuncImpArb)  "<JA_AEBfullFuncImpArb>"
  Bus Selector/1 -> BrkFumcActiveCondCalc/1(AEBpartFuncImpArb)  "<AEBpartFuncImpArb>"
  BrkFuncImpArbCalc/3(AEBfullFuncImpArb) -> Bus Creator5/3  "AEBfullFuncImpArb"
  BrkFuncImpArbCalc/8(FCTBFuncImpArb) -> Bus Creator5/8  "FCTBFuncImpArb"
  BrkFuncImpArbCalc/6(pedAEBfullFuncImpArb) -> Bus Creator5/6  "pedAEBfullFuncImpArb"
  BrkFuncImpArbCalc/5(cycAEBfullFuncImpArb) -> Bus Creator5/5  "cycAEBfullFuncImpArb"
  BrkFuncImpArbCalc/1(AEBpartFuncImpArb) -> Bus Creator5/1  "AEBpartFuncImpArb"
  BrkFuncImpArbCalc/4(JA_vehAEBfullFuncImpArb) -> Bus Creator5/4  "JA_AEBfullFuncImpArb"
  BrkFuncImpArbCalc/7(EBAFuncImpArb) -> Bus Creator5/7  "EBAFuncImpArb"
  BrkFuncImpArbCalc/2(cycAEBpartFuncImpArb) -> Bus Creator5/2  "cycAEBpartFuncImpArb"
  Bus Creator/1 -> BrkFuncImpArbCalc/2(brkFuncImpAborCondCond), Bus Creator2/1  "brkFuncImpAborCond"
  Bus Creator4/1 -> Bus Selector1/1, Bus Selector2/1, BrkFuncImpArbCalc/1(AEBModStsArb)
  BrkFumcActiveCondCalc/1(brkFuncActvCond) -> brkFuncActvCond/1
